simulink model "neuronchainv9"  (Simulink 7.6) (continued)
== system neuronchainv9/Hebbconp1to1 == (continued)
  p18/1 -> s5/3
  p19/1 -> s5/4
  p20/1 -> s5/5
  s4/1 -> Mux/3
  s1/1 -> Mux/1
  s2/1 -> Mux/2
  s3/1 -> Mux/5
  s5/1 -> Mux/4
  Mux/1 -> Iu1,Iu2,Iu3,Iu4,Iu5/1
  Mux1/1 -> sij N1/1
  Mux2/1 -> sij N4/1
  Mux3/1 -> sij N3/1
  Mux4/1 -> sij N5/1
  Mux5/1 -> sij N2/1

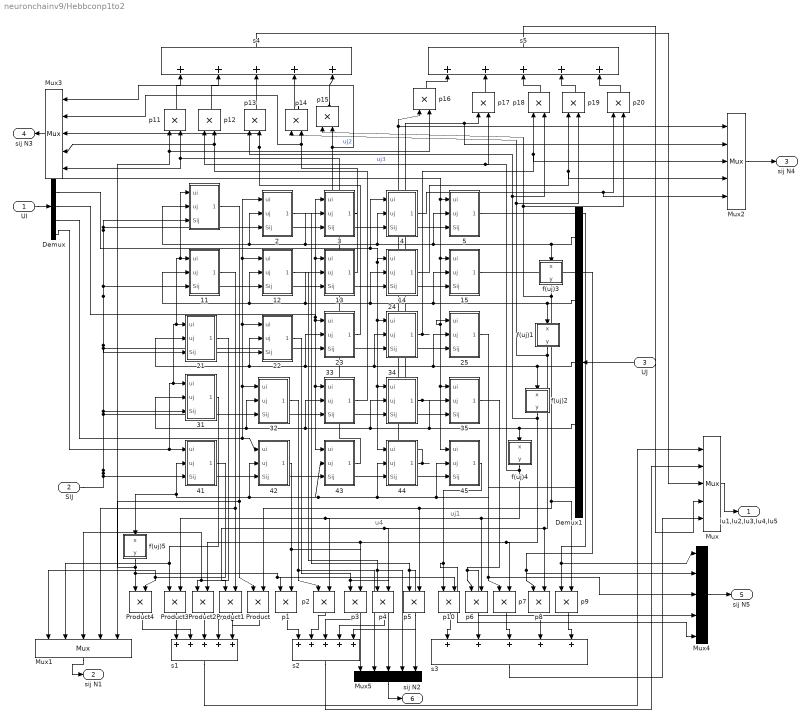
== system neuronchainv9/Hebbconp1to2 ==
  note: uj1
  note: u4
  UI : Inport
  SIJ : Inport  Port=2
  UJ : Inport  Port=3
  " " : Subsystem
  " 2" : Subsystem
  11 : Subsystem
  12 : Subsystem
  13 : Subsystem
  14 : Subsystem
  15 : Subsystem
  21 : Subsystem
  22 : Subsystem
  23 : Subsystem
  24 : Subsystem
  25 : Subsystem
  3 : Subsystem
  31 : Subsystem
  32 : Subsystem
  33 : Subsystem
  34 : Subsystem
  35 : Subsystem
  4 : Subsystem
  41 : Subsystem
  42 : Subsystem
  43 : Subsystem
  44 : Subsystem
  45 : Subsystem
  5 : Subsystem
  Demux : Demux  Outputs=5
  Demux1 : Demux  Outputs=5
  Mux{,1..5} (6 alike) : Mux  Inputs=5
  Product{,1..4} (5 alike) : Product  RndMeth=Zero
  f(uj)1 : Subsystem  TreatAsAtomicUnit=on  mask{slope=sigslopef, offset=0.5}
      mask help: Created by Han, Cheol
      mask Display: plot([10 15 20 25 30 35 40 45 50], [0 0.01 0.1 0.2 0.5 0.8 0.9 0.99 1])
  f(uj)2 : Subsystem  TreatAsAtomicUnit=on  mask{slope=sigslopef, offset=0.5}
      mask help: Created by Han, Cheol
      mask Display: plot([10 15 20 25 30 35 40 45 50], [0 0.01 0.1 0.2 0.5 0.8 0.9 0.99 1])
  f(uj)3 : Subsystem  TreatAsAtomicUnit=on  mask{slope=sigslopef, offset=0.5}
      mask help: Created by Han, Cheol
      mask Display: plot([10 15 20 25 30 35 40 45 50], [0 0.01 0.1 0.2 0.5 0.8 0.9 0.99 1])
  f(uj)4 : Subsystem  TreatAsAtomicUnit=on  mask{slope=sigslopef, offset=0.5}
      mask help: Created by Han, Cheol
      mask Display: plot([10 15 20 25 30 35 40 45 50], [0 0.01 0.1 0.2 0.5 0.8 0.9 0.99 1])
  f(uj)5 : Subsystem  TreatAsAtomicUnit=on  mask{slope=sigslopef, offset=0.5}
      mask help: Created by Han, Cheol
      mask Display: plot([10 15 20 25 30 35 40 45 50], [0 0.01 0.1 0.2 0.5 0.8 0.9 0.99 1])
  p{1..20} (20 alike) : Product  RndMeth=Zero
  s{1..5} (5 alike) : Sum  Inputs=+++++
  Iu1,Iu2,Iu3,Iu4,Iu5 : Outport
  sij N1 : Outport  Port=2
  sij N4 : Outport  Port=3
  sij N3 : Outport  Port=4
  sij N5 : Outport  Port=5
  sij N2 : Outport  Port=6
  wires:
  Demux/1 -> 15/1(ui), 45/1(ui), 35/1(ui), 25/1(ui), 5/1(ui)
  Demux/2 -> 4/1(ui), 44/1(ui), 34/1(ui), 24/1(ui), 14/1(ui)
  Demux/3 -> 43/1(ui), 33/1(ui), 23/1(ui), 13/1(ui), 3/1(ui)
  Demux/4 -> 42/1(ui), 32/1(ui), 22/1(ui), 12/1(ui), " 2"/1(ui)
  Demux/5 -> 41/1(ui), 31/1(ui), 21/1(ui), 11/1(ui), " "/1(ui)
  Demux1/1 -> " "/2(uj), " 2"/2(uj), 3/2(uj), 4/2(uj), 5/2(uj), f(uj)3/1(x)
  Demux1/2 -> 11/2(uj), 12/2(uj), 13/2(uj), 14/2(uj), 15/2(uj), f(uj)1/1(x)
  Demux1/3 -> 25/2(uj), 21/2(uj), 22/2(uj), 23/2(uj), 24/2(uj), f(uj)2/1(x)
  Demux1/4 -> 31/2(uj), 32/2(uj), 33/2(uj), 34/2(uj), 35/2(uj), f(uj)4/1(x)
  Demux1/5 -> 42/2(uj), 41/2(uj), f(uj)5/1(x), 43/2(uj), 44/2(uj), 45/2(uj)
  " "/1(Out1) -> Product/1, Mux1/5
  11/1(Out1) -> Product1/2, Mux1/4
  41/1(Out1) -> Product4/2, Mux1/1
  21/1(Out1) -> Product2/1, Mux1/3
  31/1(Out1) -> Product3/1, Mux1/2
  f(uj)3/1(y) -> Product/2, p5/2, p9/2, p15/1, p20/2
  f(uj)1/1(y) -> Product1/1, p4/2, p8/2, p14/1, p19/2  "uj2"
  f(uj)2/1(y) -> Product2/2, p3/2, p7/2, p13/1, p18/2  "uj3"
  f(uj)4/1(y) -> Product3/2, p2/2, p6/2, p17/2, p12/1
  " 2"/1(Out1) -> p5/1, Mux5/5
  12/1(Out1) -> p4/1, Mux5/4
  22/1(Out1) -> p3/1, Mux5/3
  32/1(Out1) -> p2/1, Mux5/2
  42/1(Out1) -> p1/2, Mux5/1
  f(uj)5/1(y) -> Product4/1, p1/1, p10/2, p11/1, p16/2
  5/1(Out1) -> p9/1, Mux4/1
  15/1(Out1) -> p8/1, Mux4/2
  25/1(Out1) -> p7/1, Mux4/3
  35/1(Out1) -> p6/1, Mux4/4
  45/1(Out1) -> p10/1, Mux4/5
  3/1(Out1) -> p15/2, Mux3/1
  13/1(Out1) -> p14/2, Mux3/2
  23/1(Out1) -> p13/2, Mux3/3
  33/1(Out1) -> p12/2, Mux3/4
  43/1(Out1) -> p11/2, Mux3/5
  p15/1 -> s4/5
  p11/1 -> s4/1
  p12/1 -> s4/2
  p13/1 -> s4/3
  p14/1 -> s4/4
  UI/1 -> Demux/1
  4/1(Out1) -> p20/1, Mux2/5
  SIJ/1 -> 41/3(Sij), 21/3(Sij), 11/3(Sij), " "/3(Sij), " 2"/3(Sij), 3/3(Sij), 4/3(Sij), 5/3(Sij), 13/3(Sij), 14/3(Sij), 15/3(Sij), 12/3(Sij), 22/3(Sij), 23/3(Sij), 24/3(Sij), 25/3(Sij), 31/3(Sij), 33/3(Sij), 34/3(Sij), 35/3(Sij), 32/3(Sij), 42/3(Sij), 43/3(Sij), 44/3(Sij), 45/3(Sij)
  14/1(Out1) -> p19/1, Mux2/4
  24/1(Out1) -> p18/1, Mux2/3
  UJ/1 -> Demux1/1
  34/1(Out1) -> p17/1, Mux2/2
  44/1(Out1) -> p16/1, Mux2/1
  Product/1 -> s1/5
  Product1/1 -> s1/4
  Product2/1 -> s1/3
  Product3/1 -> s1/2
  Product4/1 -> s1/1
  p1/1 -> s2/1
  p10/1 -> s3/1
  p2/1 -> s2/2
  p3/1 -> s2/3
  p4/1 -> s2/4
  p5/1 -> s2/5
  p6/1 -> s3/2
  p7/1 -> s3/3
  p8/1 -> s3/4
  p9/1 -> s3/5
  p16/1 -> s5/1
  p17/1 -> s5/2
  p18/1 -> s5/3
  p19/1 -> s5/4
  p20/1 -> s5/5
  s4/1 -> Mux/3
  s1/1 -> Mux/1
  s2/1 -> Mux/2
  s3/1 -> Mux/5
  s5/1 -> Mux/4
  Mux/1 -> Iu1,Iu2,Iu3,Iu4,Iu5/1
  Mux1/1 -> sij N1/1
  Mux2/1 -> sij N4/1
  Mux3/1 -> sij N3/1
  Mux4/1 -> sij N5/1
  Mux5/1 -> sij N2/1

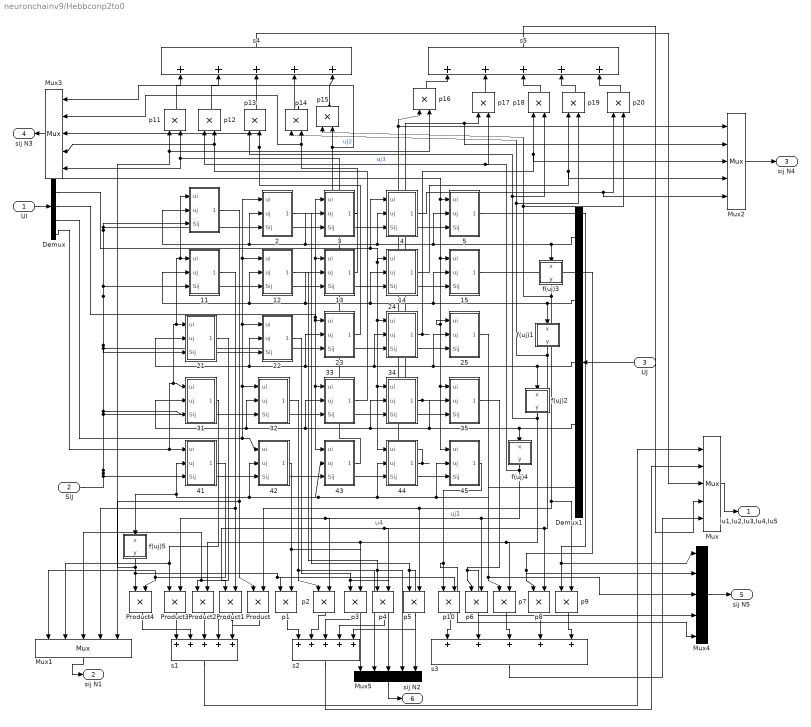
== system neuronchainv9/Hebbconp2to0 ==
  note: uj1
  note: u4
  UI : Inport
  SIJ : Inport  Port=2
  UJ : Inport  Port=3
  " " : Subsystem
  " 2" : Subsystem
  11 : Subsystem
  12 : Subsystem
  13 : Subsystem
  14 : Subsystem
  15 : Subsystem
  21 : Subsystem
  22 : Subsystem
  23 : Subsystem
  24 : Subsystem
  25 : Subsystem
  3 : Subsystem
  31 : Subsystem
  32 : Subsystem
  33 : Subsystem
  34 : Subsystem
  35 : Subsystem
  4 : Subsystem
  41 : Subsystem
  42 : Subsystem
  43 : Subsystem
  44 : Subsystem
  45 : Subsystem
  5 : Subsystem
  Demux : Demux  Outputs=5
  Demux1 : Demux  Outputs=5
  Mux{,1..5} (6 alike) : Mux  Inputs=5
  Product{,1..4} (5 alike) : Product  RndMeth=Zero
  f(uj)1 : Subsystem  TreatAsAtomicUnit=on  mask{slope=sigslopef, offset=0.5}
      mask help: Created by Han, Cheol
      mask Display: plot([10 15 20 25 30 35 40 45 50], [0 0.01 0.1 0.2 0.5 0.8 0.9 0.99 1])
  f(uj)2 : Subsystem  TreatAsAtomicUnit=on  mask{slope=sigslopef, offset=0.5}
      mask help: Created by Han, Cheol
      mask Display: plot([10 15 20 25 30 35 40 45 50], [0 0.01 0.1 0.2 0.5 0.8 0.9 0.99 1])
  f(uj)3 : Subsystem  TreatAsAtomicUnit=on  mask{slope=sigslopef, offset=0.5}
      mask help: Created by Han, Cheol
      mask Display: plot([10 15 20 25 30 35 40 45 50], [0 0.01 0.1 0.2 0.5 0.8 0.9 0.99 1])
  f(uj)4 : Subsystem  TreatAsAtomicUnit=on  mask{slope=sigslopef, offset=0.5}
      mask help: Created by Han, Cheol
      mask Display: plot([10 15 20 25 30 35 40 45 50], [0 0.01 0.1 0.2 0.5 0.8 0.9 0.99 1])
  f(uj)5 : Subsystem  TreatAsAtomicUnit=on  mask{slope=sigslopef, offset=0.5}
      mask help: Created by Han, Cheol
      mask Display: plot([10 15 20 25 30 35 40 45 50], [0 0.01 0.1 0.2 0.5 0.8 0.9 0.99 1])
  p{1..20} (20 alike) : Product  RndMeth=Zero
  s{1..5} (5 alike) : Sum  Inputs=+++++
  Iu1,Iu2,Iu3,Iu4,Iu5 : Outport
  sij N1 : Outport  Port=2
  sij N4 : Outport  Port=3
  sij N3 : Outport  Port=4
  sij N5 : Outport  Port=5
  sij N2 : Outport  Port=6
  wires:
  Demux/1 -> 15/1(ui), 45/1(ui), 35/1(ui), 25/1(ui), 5/1(ui)
  Demux/2 -> 4/1(ui), 44/1(ui), 34/1(ui), 24/1(ui), 14/1(ui)
  Demux/3 -> 43/1(ui), 33/1(ui), 23/1(ui), 13/1(ui), 3/1(ui)
  Demux/4 -> 42/1(ui), 32/1(ui), 22/1(ui), 12/1(ui), " 2"/1(ui)
  Demux/5 -> 41/1(ui), 31/1(ui), 21/1(ui), 11/1(ui), " "/1(ui)
  Demux1/1 -> " "/2(uj), " 2"/2(uj), 3/2(uj), 4/2(uj), 5/2(uj), f(uj)3/1(x)
  Demux1/2 -> 11/2(uj), 12/2(uj), 13/2(uj), 14/2(uj), 15/2(uj), f(uj)1/1(x)
  Demux1/3 -> 25/2(uj), 21/2(uj), 22/2(uj), 23/2(uj), 24/2(uj), f(uj)2/1(x)
  Demux1/4 -> 31/2(uj), 32/2(uj), 33/2(uj), 34/2(uj), 35/2(uj), f(uj)4/1(x)
  Demux1/5 -> 42/2(uj), 41/2(uj), f(uj)5/1(x), 43/2(uj), 44/2(uj), 45/2(uj)
  " "/1(Out1) -> Product/1, Mux1/5
  11/1(Out1) -> Product1/2, Mux1/4
  41/1(Out1) -> Product4/2, Mux1/1
  21/1(Out1) -> Product2/1, Mux1/3
  31/1(Out1) -> Product3/1, Mux1/2
  f(uj)3/1(y) -> Product/2, p5/2, p9/2, p15/1, p20/2
  f(uj)1/1(y) -> Product1/1, p4/2, p8/2, p14/1, p19/2  "uj2"
  f(uj)2/1(y) -> Product2/2, p3/2, p7/2, p13/1, p18/2  "uj3"
  f(uj)4/1(y) -> Product3/2, p2/2, p6/2, p17/2, p12/1
  " 2"/1(Out1) -> p5/1, Mux5/5
  12/1(Out1) -> p4/1, Mux5/4
  22/1(Out1) -> p3/1, Mux5/3
  32/1(Out1) -> p2/1, Mux5/2
  42/1(Out1) -> p1/2, Mux5/1
  f(uj)5/1(y) -> Product4/1, p1/1, p10/2, p11/1, p16/2
  5/1(Out1) -> p9/1, Mux4/1
  15/1(Out1) -> p8/1, Mux4/2
  25/1(Out1) -> p7/1, Mux4/3
  35/1(Out1) -> p6/1, Mux4/4
  45/1(Out1) -> p10/1, Mux4/5
  3/1(Out1) -> p15/2, Mux3/1
  13/1(Out1) -> p14/2, Mux3/2
  23/1(Out1) -> p13/2, Mux3/3
  33/1(Out1) -> p12/2, Mux3/4
  43/1(Out1) -> p11/2, Mux3/5
  p15/1 -> s4/5
  p11/1 -> s4/1
  p12/1 -> s4/2
  p13/1 -> s4/3
  p14/1 -> s4/4
  UI/1 -> Demux/1
  4/1(Out1) -> p20/1, Mux2/5
  SIJ/1 -> 41/3(Sij), 21/3(Sij), 11/3(Sij), " "/3(Sij), " 2"/3(Sij), 3/3(Sij), 4/3(Sij), 5/3(Sij), 13/3(Sij), 14/3(Sij), 15/3(Sij), 12/3(Sij), 22/3(Sij), 23/3(Sij), 24/3(Sij), 25/3(Sij), 31/3(Sij), 33/3(Sij), 34/3(Sij), 35/3(Sij), 32/3(Sij), 42/3(Sij), 43/3(Sij), 44/3(Sij), 45/3(Sij)
  14/1(Out1) -> p19/1, Mux2/4
  24/1(Out1) -> p18/1, Mux2/3
  UJ/1 -> Demux1/1
  34/1(Out1) -> p17/1, Mux2/2
  44/1(Out1) -> p16/1, Mux2/1
  Product/1 -> s1/5
  Product1/1 -> s1/4
  Product2/1 -> s1/3
  Product3/1 -> s1/2
  Product4/1 -> s1/1
  p1/1 -> s2/1
  p10/1 -> s3/1
  p2/1 -> s2/2
  p3/1 -> s2/3
  p4/1 -> s2/4
  p5/1 -> s2/5
  p6/1 -> s3/2
  p7/1 -> s3/3
  p8/1 -> s3/4
  p9/1 -> s3/5
  p16/1 -> s5/1
  p17/1 -> s5/2
  p18/1 -> s5/3
  p19/1 -> s5/4
  p20/1 -> s5/5
  s4/1 -> Mux/3
  s1/1 -> Mux/1
  s2/1 -> Mux/2
  s3/1 -> Mux/5
  s5/1 -> Mux/4
  Mux/1 -> Iu1,Iu2,Iu3,Iu4,Iu5/1
  Mux1/1 -> sij N1/1
  Mux2/1 -> sij N4/1
  Mux3/1 -> sij N3/1
  Mux4/1 -> sij N5/1
  Mux5/1 -> sij N2/1

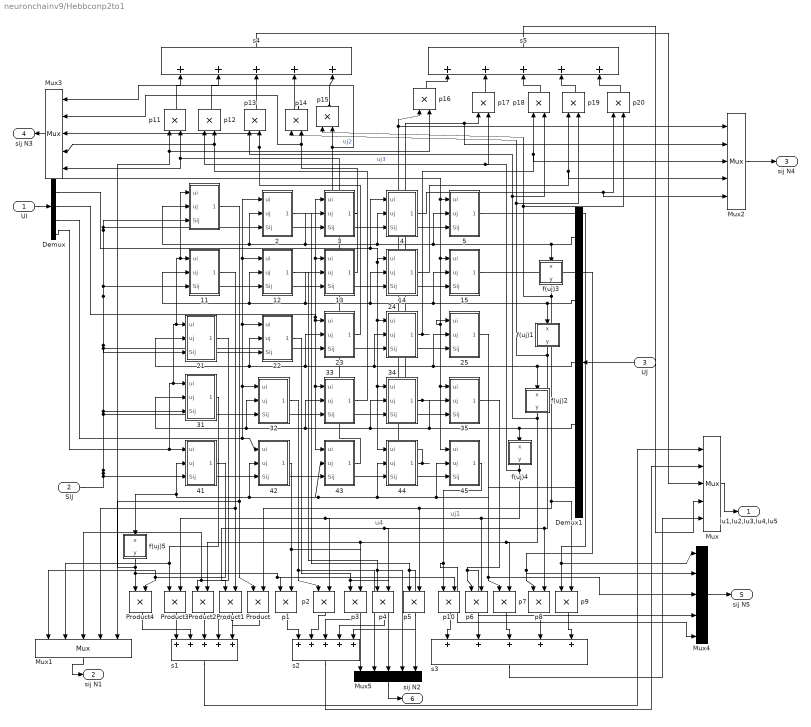
== system neuronchainv9/Hebbconp2to1 ==
  note: uj1
  note: u4
  UI : Inport
  SIJ : Inport  Port=2
  UJ : Inport  Port=3
  " " : Subsystem
  " 2" : Subsystem
  11 : Subsystem
  12 : Subsystem
  13 : Subsystem
  14 : Subsystem
  15 : Subsystem
  21 : Subsystem
  22 : Subsystem
  23 : Subsystem
  24 : Subsystem
  25 : Subsystem
  3 : Subsystem
  31 : Subsystem
  32 : Subsystem
  33 : Subsystem
  34 : Subsystem
  35 : Subsystem
  4 : Subsystem
  41 : Subsystem
  42 : Subsystem
  43 : Subsystem
  44 : Subsystem
  45 : Subsystem
  5 : Subsystem
  Demux : Demux  Outputs=5
  Demux1 : Demux  Outputs=5
  Mux{,1..5} (6 alike) : Mux  Inputs=5
  Product{,1..4} (5 alike) : Product  RndMeth=Zero
  f(uj)1 : Subsystem  TreatAsAtomicUnit=on  mask{slope=sigslopef, offset=0.5}
      mask help: Created by Han, Cheol
      mask Display: plot([10 15 20 25 30 35 40 45 50], [0 0.01 0.1 0.2 0.5 0.8 0.9 0.99 1])
  f(uj)2 : Subsystem  TreatAsAtomicUnit=on  mask{slope=sigslopef, offset=0.5}
      mask help: Created by Han, Cheol
      mask Display: plot([10 15 20 25 30 35 40 45 50], [0 0.01 0.1 0.2 0.5 0.8 0.9 0.99 1])
  f(uj)3 : Subsystem  TreatAsAtomicUnit=on  mask{slope=sigslopef, offset=0.5}
      mask help: Created by Han, Cheol
      mask Display: plot([10 15 20 25 30 35 40 45 50], [0 0.01 0.1 0.2 0.5 0.8 0.9 0.99 1])
  f(uj)4 : Subsystem  TreatAsAtomicUnit=on  mask{slope=sigslopef, offset=0.5}
      mask help: Created by Han, Cheol
      mask Display: plot([10 15 20 25 30 35 40 45 50], [0 0.01 0.1 0.2 0.5 0.8 0.9 0.99 1])
  f(uj)5 : Subsystem  TreatAsAtomicUnit=on  mask{slope=sigslopef, offset=0.5}
      mask help: Created by Han, Cheol
      mask Display: plot([10 15 20 25 30 35 40 45 50], [0 0.01 0.1 0.2 0.5 0.8 0.9 0.99 1])
  p{1..20} (20 alike) : Product  RndMeth=Zero
  s{1..5} (5 alike) : Sum  Inputs=+++++
  Iu1,Iu2,Iu3,Iu4,Iu5 : Outport
  sij N1 : Outport  Port=2
  sij N4 : Outport  Port=3
  sij N3 : Outport  Port=4
  sij N5 : Outport  Port=5
  sij N2 : Outport  Port=6
  wires:
  Demux/1 -> 15/1(ui), 45/1(ui), 35/1(ui), 25/1(ui), 5/1(ui)
  Demux/2 -> 4/1(ui), 44/1(ui), 34/1(ui), 24/1(ui), 14/1(ui)
  Demux/3 -> 43/1(ui), 33/1(ui), 23/1(ui), 13/1(ui), 3/1(ui)
  Demux/4 -> 42/1(ui), 32/1(ui), 22/1(ui), 12/1(ui), " 2"/1(ui)
  Demux/5 -> 41/1(ui), 31/1(ui), 21/1(ui), 11/1(ui), " "/1(ui)
  Demux1/1 -> " "/2(uj), " 2"/2(uj), 3/2(uj), 4/2(uj), 5/2(uj), f(uj)3/1(x)
  Demux1/2 -> 11/2(uj), 12/2(uj), 13/2(uj), 14/2(uj), 15/2(uj), f(uj)1/1(x)
  Demux1/3 -> 25/2(uj), 21/2(uj), 22/2(uj), 23/2(uj), 24/2(uj), f(uj)2/1(x)
  Demux1/4 -> 31/2(uj), 32/2(uj), 33/2(uj), 34/2(uj), 35/2(uj), f(uj)4/1(x)
  Demux1/5 -> 42/2(uj), 41/2(uj), f(uj)5/1(x), 43/2(uj), 44/2(uj), 45/2(uj)
  " "/1(Out1) -> Product/1, Mux1/5
  11/1(Out1) -> Product1/2, Mux1/4
  41/1(Out1) -> Product4/2, Mux1/1
  21/1(Out1) -> Product2/1, Mux1/3
  31/1(Out1) -> Product3/1, Mux1/2
  f(uj)3/1(y) -> Product/2, p5/2, p9/2, p15/1, p20/2
  f(uj)1/1(y) -> Product1/1, p4/2, p8/2, p14/1, p19/2  "uj2"
  f(uj)2/1(y) -> Product2/2, p3/2, p7/2, p13/1, p18/2  "uj3"
  f(uj)4/1(y) -> Product3/2, p2/2, p6/2, p17/2, p12/1
  " 2"/1(Out1) -> p5/1, Mux5/5
  12/1(Out1) -> p4/1, Mux5/4
  22/1(Out1) -> p3/1, Mux5/3
  32/1(Out1) -> p2/1, Mux5/2
  42/1(Out1) -> p1/2, Mux5/1
  f(uj)5/1(y) -> Product4/1, p1/1, p10/2, p11/1, p16/2
  5/1(Out1) -> p9/1, Mux4/1
  15/1(Out1) -> p8/1, Mux4/2
  25/1(Out1) -> p7/1, Mux4/3
  35/1(Out1) -> p6/1, Mux4/4
  45/1(Out1) -> p10/1, Mux4/5
  3/1(Out1) -> p15/2, Mux3/1
  13/1(Out1) -> p14/2, Mux3/2
  23/1(Out1) -> p13/2, Mux3/3
  33/1(Out1) -> p12/2, Mux3/4
  43/1(Out1) -> p11/2, Mux3/5
  p15/1 -> s4/5
  p11/1 -> s4/1
  p12/1 -> s4/2
  p13/1 -> s4/3
  p14/1 -> s4/4
  UI/1 -> Demux/1
  4/1(Out1) -> p20/1, Mux2/5
  SIJ/1 -> 41/3(Sij), 21/3(Sij), 11/3(Sij), " "/3(Sij), " 2"/3(Sij), 3/3(Sij), 4/3(Sij), 5/3(Sij), 13/3(Sij), 14/3(Sij), 15/3(Sij), 12/3(Sij), 22/3(Sij), 23/3(Sij), 24/3(Sij), 25/3(Sij), 31/3(Sij), 33/3(Sij), 34/3(Sij), 35/3(Sij), 32/3(Sij), 42/3(Sij), 43/3(Sij), 44/3(Sij), 45/3(Sij)
  14/1(Out1) -> p19/1, Mux2/4
  24/1(Out1) -> p18/1, Mux2/3
  UJ/1 -> Demux1/1
  34/1(Out1) -> p17/1, Mux2/2
  44/1(Out1) -> p16/1, Mux2/1
  Product/1 -> s1/5
  Product1/1 -> s1/4
  Product2/1 -> s1/3
  Product3/1 -> s1/2
  Product4/1 -> s1/1
  p1/1 -> s2/1
  p10/1 -> s3/1
  p2/1 -> s2/2
  p3/1 -> s2/3
  p4/1 -> s2/4
  p5/1 -> s2/5
  p6/1 -> s3/2
  p7/1 -> s3/3
  p8/1 -> s3/4
  p9/1 -> s3/5
  p16/1 -> s5/1
  p17/1 -> s5/2
  p18/1 -> s5/3
  p19/1 -> s5/4
  p20/1 -> s5/5
  s4/1 -> Mux/3
  s1/1 -> Mux/1
  s2/1 -> Mux/2
  s3/1 -> Mux/5
  s5/1 -> Mux/4
  Mux/1 -> Iu1,Iu2,Iu3,Iu4,Iu5/1
  Mux1/1 -> sij N1/1
  Mux2/1 -> sij N4/1
  Mux3/1 -> sij N3/1
  Mux4/1 -> sij N5/1
  Mux5/1 -> sij N2/1

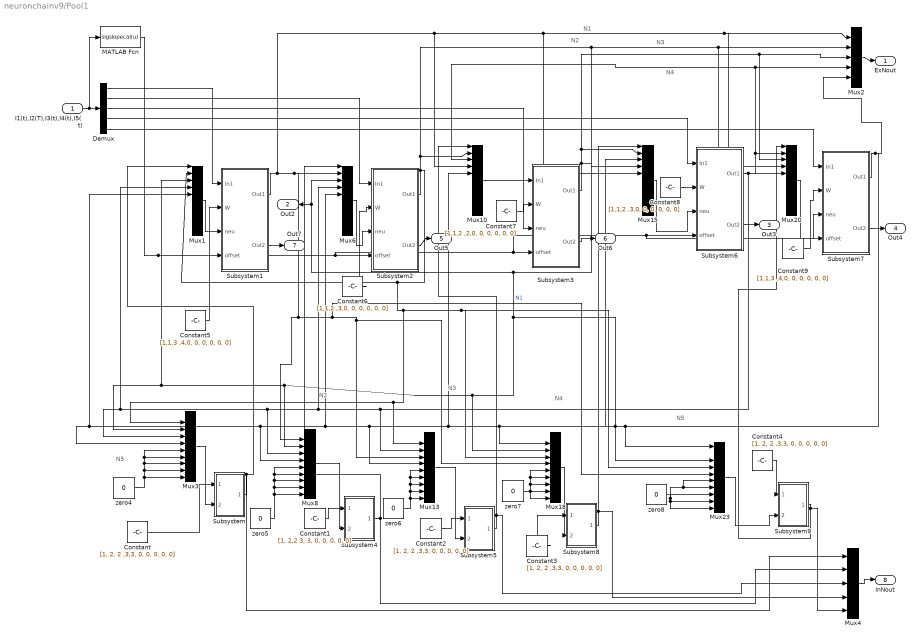
== system neuronchainv9/Pool1 ==
  note: N2
  note: N3
  note: N4
  note: N5
  note: N5
  note: N1
  note: N2
  note: N3
  note: N4
  I1(t),I2(T),I3(t),I4(t),I5(t) : Inport
  Constant{,2..4} (4 alike) : Constant  Value=[1, 2, 2 ,3,3, 0, 0, 0, 0, 0]
  Constant1 : Constant  Value=[1, 2,2 3, 3, 0, 0, 0, 0, 0]
  Constant5 : Constant  Value=[1,1,3 ,4,0, 0, 0, 0, 0, 0]
  Constant6 : Constant  Value=[1,1,2 ,3,0, 0, 0, 0, 0, 0]
  Constant7 : Constant  Value=[1,1,2 ,2,0, 0, 0, 0, 0, 0]
  Constant8 : Constant  Value=[1,1,2 ,3,0, 0, 0, 0, 0, 0]
  Constant9 : Constant  Value=[1,1,3 ,4,0, 0, 0, 0, 0, 0]
  Demux : Demux  Outputs=5
  MATLAB Fcn : MATLABFcn  MATLABFcn=sigslopecal(u)
  Mux{1,6,10,15,20} (5 alike) : Mux  Inputs=11
  Mux{3,8,13,18,23} (5 alike) : Mux  Inputs=10
  Mux2 : Mux  Inputs=5
  Mux4 : Mux  Inputs=5
  Subsystem : Subsystem
  Subsystem1 : Subsystem
  Subsystem2 : Subsystem
  Subsystem3 : Subsystem
  Subsystem4 : Subsystem
  Subsystem5 : Subsystem
  Subsystem6 : Subsystem
  Subsystem7 : Subsystem
  Subsystem8 : Subsystem
  Subsystem9 : Subsystem
  zero{4..8} (5 alike) : Constant  Value=0
  ExNout : Outport
  Out2 : Outport  Port=2
  Out3 : Outport  Port=3
  Out4 : Outport  Port=4
  Out5 : Outport  Port=5
  Out6 : Outport  Port=6
  Out7 : Outport  Port=7
  InNout : Outport  Port=8
  wires:
  zero8/1 -> Mux23/6, Mux23/7, Mux23/8, Mux23/9, Mux23/10
  Subsystem1/2(Out2) -> Out7/1
  Subsystem3/2(Out2) -> Out6/1
  Subsystem2/2(Out2) -> Out5/1
  Subsystem7/2(Out2) -> Out4/1
  Subsystem6/2(Out2) -> Out3/1
  zero7/1 -> Mux18/10, Mux18/9, Mux18/8, Mux18/7, Mux18/6
  zero6/1 -> Mux13/10, Mux13/9, Mux13/8, Mux13/7, Mux13/6
  zero5/1 -> Mux8/10, Mux8/9, Mux8/8, Mux8/7, Mux8/6
  zero4/1 -> Mux3/10, Mux3/9, Mux3/8, Mux3/7, Mux3/6
  ? -> Mux6/10, Mux6/9, Mux6/8, Mux6/7, Mux6/6, Mux6/11
  ? -> Mux10/11, Mux10/10, Mux10/9, Mux10/8, Mux10/7, Mux10/6
  Constant7/1 -> Subsystem3/2(W)
  ? -> Mux15/11, Mux15/10, Mux15/9, Mux15/8, Mux15/7, Mux15/6
  ? -> Mux20/11, Mux20/10, Mux20/9, Mux20/8, Mux20/7, Mux20/6
  Mux15/1 -> Subsystem6/3(neu)
  Constant8/1 -> Subsystem6/2(W)
  Constant4/1 -> Subsystem9/1(port1)
  Constant9/1 -> Subsystem7/2(W)
  Subsystem1/1(Out1) -> Mux6/2, Mux23/5, Mux18/5, Mux13/4, Mux8/2, Mux10/4, Mux15/5, Mux2/1, Mux20/5  "N1"
  Subsystem2/1(Out1) -> Mux1/2, Mux23/4, Mux18/4, Mux13/2, Mux3/2, Mux10/2, Mux15/4, Mux2/2, Mux20/4
  Subsystem3/1(Out1) -> Mux2/3, Mux20/3, Mux15/2, Out2/1, Mux6/3, Mux23/3, Mux18/3, Mux1/3, Mux3/3, Mux8/3
  Subsystem6/1(Out1) -> Mux20/2, Mux2/4, Mux10/3, Mux13/3, Mux8/4, Mux3/4, Mux1/4, Mux6/4
  Subsystem7/1(Out1) -> Mux2/5, Mux15/3, Mux18/2, Mux13/5, Mux8/5, Mux3/5, Mux1/5, Mux6/5, Mux10/5, Mux23/1
  Mux3/1 -> Subsystem/2(port2)
  Mux8/1 -> Subsystem4/2(port2)
  Mux13/1 -> Subsystem5/2(port2)
  Mux18/1 -> Subsystem8/2(port2)
  Mux23/1 -> Subsystem9/2(port2)
  Subsystem/1(Out2) -> Mux4/1, Mux1/1
  Subsystem4/1(Out2) -> Mux4/2, Mux6/1
  Subsystem5/1(Out2) -> Mux4/3, Mux10/1
  Subsystem8/1(Out2) -> Mux4/4, Mux15/1
  Subsystem9/1(Out2) -> Mux20/1, Mux4/5
  Mux1/1 -> Subsystem1/3(neu)
  Mux6/1 -> Subsystem2/3(neu)
  Mux10/1 -> Subsystem3/3(neu)
  Mux20/1 -> Subsystem7/3(neu)
  Constant/1 -> Subsystem/1(port1)
  Constant1/1 -> Subsystem4/1(port1)
  Constant2/1 -> Subsystem5/1(port1)
  Constant3/1 -> Subsystem8/1(port1)
  Constant5/1 -> Subsystem1/2(W)
  Constant6/1 -> Subsystem2/2(W)
  I1(t),I2(T),I3(t),I4(t),I5(t)/1 -> Demux/1, MATLAB Fcn/1
  Demux/1 -> Subsystem1/1(In1)
  Demux/2 -> Subsystem2/1(In1)
  Demux/3 -> Subsystem3/1(In1)
  Demux/4 -> Subsystem6/1(In1)
  Demux/5 -> Subsystem7/1(In1)
  Mux2/1 -> ExNout/1
  Mux4/1 -> InNout/1
  ? -> Mux1/10, Mux1/11, Mux1/6, Mux1/7, Mux1/8, Mux1/9
  MATLAB Fcn/1 -> Subsystem1/4(offset), Subsystem2/4(offset), Subsystem3/4(offset), Subsystem6/4(offset), Subsystem7/4(offset)

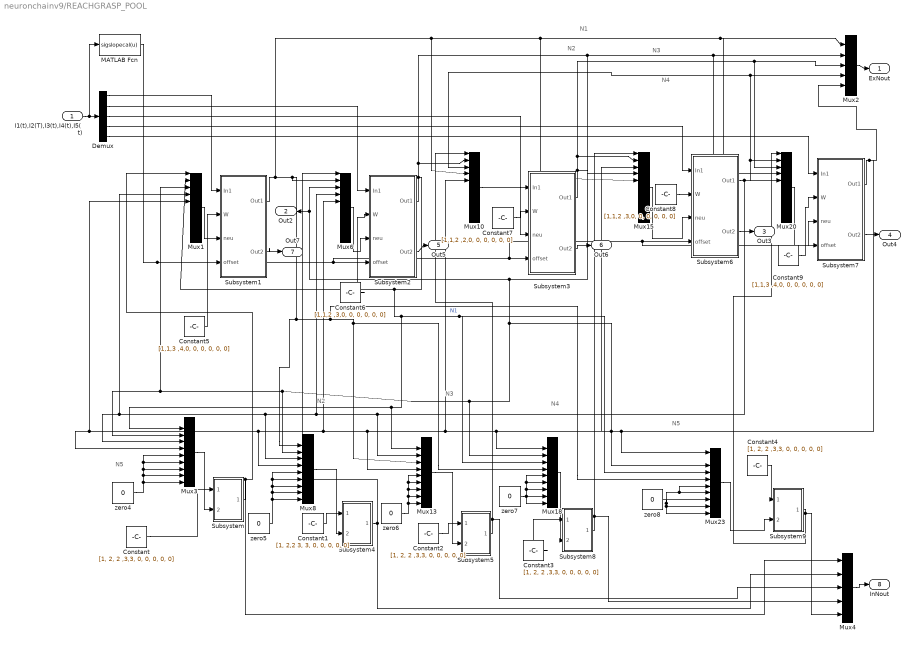
== system neuronchainv9/REACHGRASP_POOL ==
  note: N2
  note: N3
  note: N4
  note: N5
  note: N5
  note: N1
  note: N2
  note: N3
  note: N4
  I1(t),I2(T),I3(t),I4(t),I5(t) : Inport
  Constant{,2..4} (4 alike) : Constant  Value=[1, 2, 2 ,3,3, 0, 0, 0, 0, 0]
  Constant1 : Constant  Value=[1, 2,2 3, 3, 0, 0, 0, 0, 0]
  Constant5 : Constant  Value=[1,1,3 ,4,0, 0, 0, 0, 0, 0]
  Constant6 : Constant  Value=[1,1,2 ,3,0, 0, 0, 0, 0, 0]
  Constant7 : Constant  Value=[1,1,2 ,2,0, 0, 0, 0, 0, 0]
  Constant8 : Constant  Value=[1,1,2 ,3,0, 0, 0, 0, 0, 0]
  Constant9 : Constant  Value=[1,1,3 ,4,0, 0, 0, 0, 0, 0]
  Demux : Demux  Outputs=5
  MATLAB Fcn : MATLABFcn  MATLABFcn=sigslopecal(u)
  Mux{1,6,10,15,20} (5 alike) : Mux  Inputs=11
  Mux{3,8,13,18,23} (5 alike) : Mux  Inputs=10
  Mux2 : Mux  Inputs=5
  Mux4 : Mux  Inputs=5
  Subsystem : Subsystem
  Subsystem1 : Subsystem
  Subsystem2 : Subsystem
  Subsystem3 : Subsystem
  Subsystem4 : Subsystem
  Subsystem5 : Subsystem
  Subsystem6 : Subsystem
  Subsystem7 : Subsystem
  Subsystem8 : Subsystem
  Subsystem9 : Subsystem
  zero{4..8} (5 alike) : Constant  Value=0
  ExNout : Outport
  Out2 : Outport  Port=2
  Out3 : Outport  Port=3
  Out4 : Outport  Port=4
  Out5 : Outport  Port=5
  Out6 : Outport  Port=6
  Out7 : Outport  Port=7
  InNout : Outport  Port=8
  wires:
  zero8/1 -> Mux23/6, Mux23/7, Mux23/8, Mux23/9, Mux23/10
  Subsystem1/2(Out2) -> Out7/1
  Subsystem3/2(Out2) -> Out6/1
  Subsystem2/2(Out2) -> Out5/1
  Subsystem7/2(Out2) -> Out4/1
  Subsystem6/2(Out2) -> Out3/1
  zero7/1 -> Mux18/10, Mux18/9, Mux18/8, Mux18/7, Mux18/6
  zero6/1 -> Mux13/10, Mux13/9, Mux13/8, Mux13/7, Mux13/6
  zero5/1 -> Mux8/10, Mux8/9, Mux8/8, Mux8/7, Mux8/6
  zero4/1 -> Mux3/10, Mux3/9, Mux3/8, Mux3/7, Mux3/6
  ? -> Mux6/10, Mux6/9, Mux6/8, Mux6/7, Mux6/6, Mux6/11
  ? -> Mux10/11, Mux10/10, Mux10/9, Mux10/8, Mux10/7, Mux10/6
  Constant7/1 -> Subsystem3/2(W)
  ? -> Mux15/11, Mux15/10, Mux15/9, Mux15/8, Mux15/7, Mux15/6
  ? -> Mux20/11, Mux20/10, Mux20/9, Mux20/8, Mux20/7, Mux20/6
  Mux15/1 -> Subsystem6/3(neu)
  Constant8/1 -> Subsystem6/2(W)
  Constant4/1 -> Subsystem9/1(port1)
  Constant9/1 -> Subsystem7/2(W)
  Subsystem1/1(Out1) -> Mux6/2, Mux23/5, Mux18/5, Mux13/4, Mux8/2, Mux10/4, Mux15/5, Mux2/1, Mux20/5  "N1"
  Subsystem2/1(Out1) -> Mux1/2, Mux23/4, Mux18/4, Mux13/2, Mux3/2, Mux10/2, Mux15/4, Mux2/2, Mux20/4
  Subsystem3/1(Out1) -> Mux2/3, Mux20/3, Mux15/2, Out2/1, Mux6/3, Mux23/3, Mux18/3, Mux1/3, Mux3/3, Mux8/3
  Subsystem6/1(Out1) -> Mux20/2, Mux2/4, Mux10/3, Mux13/3, Mux8/4, Mux3/4, Mux1/4, Mux6/4
  Subsystem7/1(Out1) -> Mux2/5, Mux15/3, Mux18/2, Mux13/5, Mux8/5, Mux3/5, Mux1/5, Mux6/5, Mux10/5, Mux23/1
  Mux3/1 -> Subsystem/2(port2)
  Mux8/1 -> Subsystem4/2(port2)
  Mux13/1 -> Subsystem5/2(port2)
  Mux18/1 -> Subsystem8/2(port2)
  Mux23/1 -> Subsystem9/2(port2)
  Subsystem/1(Out2) -> Mux4/1, Mux1/1
  Subsystem4/1(Out2) -> Mux4/2, Mux6/1
  Subsystem5/1(Out2) -> Mux4/3, Mux10/1
  Subsystem8/1(Out2) -> Mux4/4, Mux15/1
  Subsystem9/1(Out2) -> Mux20/1, Mux4/5
  Mux1/1 -> Subsystem1/3(neu)
  Mux6/1 -> Subsystem2/3(neu)
  Mux10/1 -> Subsystem3/3(neu)
  Mux20/1 -> Subsystem7/3(neu)
  Constant/1 -> Subsystem/1(port1)
  Constant1/1 -> Subsystem4/1(port1)
  Constant2/1 -> Subsystem5/1(port1)
  Constant3/1 -> Subsystem8/1(port1)
  Constant5/1 -> Subsystem1/2(W)
  Constant6/1 -> Subsystem2/2(W)
  I1(t),I2(T),I3(t),I4(t),I5(t)/1 -> Demux/1, MATLAB Fcn/1
  Demux/1 -> Subsystem1/1(In1)
  Demux/2 -> Subsystem2/1(In1)
  Demux/3 -> Subsystem3/1(In1)
  Demux/4 -> Subsystem6/1(In1)
  Demux/5 -> Subsystem7/1(In1)
  Mux2/1 -> ExNout/1
  Mux4/1 -> InNout/1
  ? -> Mux1/10, Mux1/11, Mux1/6, Mux1/7, Mux1/8, Mux1/9
  MATLAB Fcn/1 -> Subsystem1/4(offset), Subsystem2/4(offset), Subsystem3/4(offset), Subsystem6/4(offset), Subsystem7/4(offset)

== system neuronchainv9/Subsystem ==
  Product : Product  RndMeth=Zero
  Saturation : Saturate  UpperLimit=1  LowerLimit=0
  Signal Generator : SignalGenerator  WaveForm=square  Frequency=0.25
  Sum : Sum  Inputs=|+-
  motoract vis inputs : Subsystem
  Out1 : Outport
  Out2 : Outport  Port=2
  Out3 : Outport  Port=3
  Out4 : Outport  Port=4
  wires:
  motoract vis inputs/1(Out1) -> Out1/1
  motoract vis inputs/2(Out2) -> Product/1, Sum/1, Out2/1
  Signal Generator/1 -> Product/2
  Product/1 -> Sum/2, Saturation/1
  Sum/1 -> Out4/1
  Saturation/1 -> Out3/1

== system neuronchainv9/main signal ==
  Mux : Mux  Inputs=5
  Pulse Generator{,1..4} (5 alike) : DiscretePulseGenerator  PulseType=Time based  PulseWidth=50
  Random Number{,1..4} (5 alike) : RandomNumber  Variance=vari  SampleTime=0.1
  Sum{,1..4} (5 alike) : Sum  Inputs=|++
  Out1 : Outport
  wires:
  Pulse Generator/1 -> Sum/2
  Random Number/1 -> Sum/1
  Sum/1 -> Mux/2
  Pulse Generator1/1 -> Sum1/2
  Random Number1/1 -> Sum1/1
  Sum1/1 -> Mux/1
  Pulse Generator2/1 -> Sum2/2
  Random Number2/1 -> Sum2/1
  Sum2/1 -> Mux/3
  Pulse Generator3/1 -> Sum3/2
  Random Number3/1 -> Sum3/1
  Sum3/1 -> Mux/5
  Pulse Generator4/1 -> Sum4/2
  Random Number4/1 -> Sum4/1
  Sum4/1 -> Mux/4
  Mux/1 -> Out1/1

== system neuronchainv9/main signalk ==
  Mux : Mux  Inputs=5
  Pulse Generator{,1..4} (5 alike) : DiscretePulseGenerator  PulseType=Time based  PulseWidth=50
  Random Number{,1..4} (5 alike) : RandomNumber  Variance=vari  SampleTime=0.1
  Sum{,1..4} (5 alike) : Sum  Inputs=|++
  Out1 : Outport
  wires:
  Pulse Generator/1 -> Sum/2
  Random Number/1 -> Sum/1
  Sum/1 -> Mux/2
  Pulse Generator1/1 -> Sum1/2
  Random Number1/1 -> Sum1/1
  Sum1/1 -> Mux/1
  Pulse Generator2/1 -> Sum2/2
  Random Number2/1 -> Sum2/1
  Sum2/1 -> Mux/3
  Pulse Generator3/1 -> Sum3/2
  Random Number3/1 -> Sum3/1
  Sum3/1 -> Mux/5
  Pulse Generator4/1 -> Sum4/2
  Random Number4/1 -> Sum4/1
  Sum4/1 -> Mux/4
  Mux/1 -> Out1/1

== system neuronchainv9/signalsplit1 ==
  Mux : Mux  Inputs=5
  Pulse Generator{1..5} (5 alike) : DiscretePulseGenerator  PulseType=Time based  Period=4  PulseWidth=25  PhaseDelay=0.35
  Random Number{1..5} (5 alike) : RandomNumber  Variance=vari  SampleTime=0.1
  Sum{1..5} (5 alike) : Sum  Inputs=|++
  Out1 : Outport
  wires:
  Random Number1/1 -> Sum1/1
  Pulse Generator1/1 -> Sum1/2
  Random Number2/1 -> Sum2/1
  Pulse Generator2/1 -> Sum2/2
  Random Number3/1 -> Sum3/1
  Pulse Generator3/1 -> Sum3/2
  Random Number4/1 -> Sum4/1
  Pulse Generator4/1 -> Sum4/2
  Random Number5/1 -> Sum5/1
  Pulse Generator5/1 -> Sum5/2
  Sum5/1 -> Mux/1
  Sum2/1 -> Mux/2
  Sum1/1 -> Mux/3
  Sum4/1 -> Mux/4
  Sum3/1 -> Mux/5
  Mux/1 -> Out1/1
